simulink model "preliminar"  (R2013b) (continued)
== system preliminar == (continued)
      mask init:
      | pipeline_init(gcb,'latency',latency);
      settings: AttributesFormatString=Latency = %<latency>
      mask Display:
      | port_label('input', 1, 'd');
      | port_label('output', 1, 'q');
      | disp(['Z^-', num2str(latency)]);
  pipeline35 : Subsystem  AncestorBlock=casper_library_delays/pipeline  mask(pipeline){latency=1}
      mask help:
      | An explicitly laid-out delay line to avoid mapping to SRL16s
      | for use in pipelining to help achieve timing closure.
      mask init:
      | pipeline_init(gcb,'latency',latency);
      settings: AttributesFormatString=Latency = %<latency>
      mask Display:
      | port_label('input', 1, 'd');
      | port_label('output', 1, 'q');
      | disp(['Z^-', num2str(latency)]);
  pipeline36 : Subsystem  AncestorBlock=casper_library_delays/pipeline  mask(pipeline){latency=1}
      mask help:
      | An explicitly laid-out delay line to avoid mapping to SRL16s
      | for use in pipelining to help achieve timing closure.
      mask init:
      | pipeline_init(gcb,'latency',latency);
      settings: AttributesFormatString=Latency = %<latency>
      mask Display:
      | port_label('input', 1, 'd');
      | port_label('output', 1, 'q');
      | disp(['Z^-', num2str(latency)]);
  pipeline37 : Subsystem  AncestorBlock=casper_library_delays/pipeline  mask(pipeline){latency=1}
      mask help:
      | An explicitly laid-out delay line to avoid mapping to SRL16s
      | for use in pipelining to help achieve timing closure.
      mask init:
      | pipeline_init(gcb,'latency',latency);
      settings: AttributesFormatString=Latency = %<latency>
      mask Display:
      | port_label('input', 1, 'd');
      | port_label('output', 1, 'q');
      | disp(['Z^-', num2str(latency)]);
  pipeline38 : Subsystem  AncestorBlock=casper_library_delays/pipeline  mask(pipeline){latency=1}
      mask help:
      | An explicitly laid-out delay line to avoid mapping to SRL16s
      | for use in pipelining to help achieve timing closure.
      mask init:
      | pipeline_init(gcb,'latency',latency);
      settings: AttributesFormatString=Latency = %<latency>
      mask Display:
      | port_label('input', 1, 'd');
      | port_label('output', 1, 'q');
      | disp(['Z^-', num2str(latency)]);
  pipeline39 : Subsystem  AncestorBlock=casper_library_delays/pipeline  mask(pipeline){latency=1}
      mask help:
      | An explicitly laid-out delay line to avoid mapping to SRL16s
      | for use in pipelining to help achieve timing closure.
      mask init:
      | pipeline_init(gcb,'latency',latency);
      settings: AttributesFormatString=Latency = %<latency>
      mask Display:
      | port_label('input', 1, 'd');
      | port_label('output', 1, 'q');
      | disp(['Z^-', num2str(latency)]);
  pipeline40 : Subsystem  AncestorBlock=casper_library_delays/pipeline  mask(pipeline){latency=1}
      mask help:
      | An explicitly laid-out delay line to avoid mapping to SRL16s
      | for use in pipelining to help achieve timing closure.
      mask init:
      | pipeline_init(gcb,'latency',latency);
      settings: AttributesFormatString=Latency = %<latency>
      mask Display:
      | port_label('input', 1, 'd');
      | port_label('output', 1, 'q');
      | disp(['Z^-', num2str(latency)]);
  pipeline41 : Subsystem  AncestorBlock=casper_library_delays/pipeline  mask(pipeline){latency=1}
      mask help:
      | An explicitly laid-out delay line to avoid mapping to SRL16s
      | for use in pipelining to help achieve timing closure.
      mask init:
      | pipeline_init(gcb,'latency',latency);
      settings: AttributesFormatString=Latency = %<latency>
      mask Display:
      | port_label('input', 1, 'd');
      | port_label('output', 1, 'q');
      | disp(['Z^-', num2str(latency)]);
  pipeline42 : Subsystem  AncestorBlock=casper_library_delays/pipeline  mask(pipeline){latency=1}
      mask help:
      | An explicitly laid-out delay line to avoid mapping to SRL16s
      | for use in pipelining to help achieve timing closure.
      mask init:
      | pipeline_init(gcb,'latency',latency);
      settings: AttributesFormatString=Latency = %<latency>
      mask Display:
      | port_label('input', 1, 'd');
      | port_label('output', 1, 'q');
      | disp(['Z^-', num2str(latency)]);
  pipeline43 : Subsystem  AncestorBlock=casper_library_delays/pipeline  mask(pipeline){latency=1}
      mask help:
      | An explicitly laid-out delay line to avoid mapping to SRL16s
      | for use in pipelining to help achieve timing closure.
      mask init:
      | pipeline_init(gcb,'latency',latency);
      settings: AttributesFormatString=Latency = %<latency>
      mask Display:
      | port_label('input', 1, 'd');
      | port_label('output', 1, 'q');
      | disp(['Z^-', num2str(latency)]);
  pipeline44 : Subsystem  AncestorBlock=casper_library_delays/pipeline  mask(pipeline){latency=1}
      mask help:
      | An explicitly laid-out delay line to avoid mapping to SRL16s
      | for use in pipelining to help achieve timing closure.
      mask init:
      | pipeline_init(gcb,'latency',latency);
      settings: AttributesFormatString=Latency = %<latency>
      mask Display:
      | port_label('input', 1, 'd');
      | port_label('output', 1, 'q');
      | disp(['Z^-', num2str(latency)]);
  pipeline56 : Subsystem  AncestorBlock=casper_library_delays/pipeline  mask(pipeline){latency=2}
      mask help:
      | An explicitly laid-out delay line to avoid mapping to SRL16s
      | for use in pipelining to help achieve timing closure.
      mask init:
      | pipeline_init(gcb,'latency',latency);
      settings: AttributesFormatString=Latency = %<latency>
      mask Display:
      | port_label('input', 1, 'd');
      | port_label('output', 1, 'q');
      | disp(['Z^-', num2str(latency)]);
  pipeline57 : Subsystem  AncestorBlock=casper_library_delays/pipeline  mask(pipeline){latency=2}
      mask help:
      | An explicitly laid-out delay line to avoid mapping to SRL16s
      | for use in pipelining to help achieve timing closure.
      mask init:
      | pipeline_init(gcb,'latency',latency);
      settings: AttributesFormatString=Latency = %<latency>
      mask Display:
      | port_label('input', 1, 'd');
      | port_label('output', 1, 'q');
      | disp(['Z^-', num2str(latency)]);
  pipeline58 : Subsystem  AncestorBlock=casper_library_delays/pipeline  mask(pipeline){latency=1}
      mask help:
      | An explicitly laid-out delay line to avoid mapping to SRL16s
      | for use in pipelining to help achieve timing closure.
      mask init:
      | pipeline_init(gcb,'latency',latency);
      settings: AttributesFormatString=Latency = %<latency>
      mask Display:
      | port_label('input', 1, 'd');
      | port_label('output', 1, 'q');
      | disp(['Z^-', num2str(latency)]);
  pipeline59 : Subsystem  AncestorBlock=casper_library_delays/pipeline  mask(pipeline){latency=1}
      mask help:
      | An explicitly laid-out delay line to avoid mapping to SRL16s
      | for use in pipelining to help achieve timing closure.
      mask init:
      | pipeline_init(gcb,'latency',latency);
      settings: AttributesFormatString=Latency = %<latency>
      mask Display:
      | port_label('input', 1, 'd');
      | port_label('output', 1, 'q');
      | disp(['Z^-', num2str(latency)]);
  pipeline60 : Subsystem  AncestorBlock=casper_library_delays/pipeline  mask(pipeline){latency=1}
      mask help:
      | An explicitly laid-out delay line to avoid mapping to SRL16s
      | for use in pipelining to help achieve timing closure.
      mask init:
      | pipeline_init(gcb,'latency',latency);
      settings: AttributesFormatString=Latency = %<latency>
      mask Display:
      | port_label('input', 1, 'd');
      | port_label('output', 1, 'q');
      | disp(['Z^-', num2str(latency)]);
  pipeline61 : Subsystem  AncestorBlock=casper_library_delays/pipeline  mask(pipeline){latency=2}
      mask help:
      | An explicitly laid-out delay line to avoid mapping to SRL16s
      | for use in pipelining to help achieve timing closure.
      mask init:
      | pipeline_init(gcb,'latency',latency);
      settings: AttributesFormatString=Latency = %<latency>
      mask Display:
      | port_label('input', 1, 'd');
      | port_label('output', 1, 'q');
      | disp(['Z^-', num2str(latency)]);
  pipeline62 : Subsystem  AncestorBlock=casper_library_delays/pipeline  mask(pipeline){latency=2}
      mask help:
      | An explicitly laid-out delay line to avoid mapping to SRL16s
      | for use in pipelining to help achieve timing closure.
      mask init:
      | pipeline_init(gcb,'latency',latency);
      settings: AttributesFormatString=Latency = %<latency>
      mask Display:
      | port_label('input', 1, 'd');
      | port_label('output', 1, 'q');
      | disp(['Z^-', num2str(latency)]);
  pipeline63 : Subsystem  AncestorBlock=casper_library_delays/pipeline  mask(pipeline){latency=1}
      mask help:
      | An explicitly laid-out delay line to avoid mapping to SRL16s
      | for use in pipelining to help achieve timing closure.
      mask init:
      | pipeline_init(gcb,'latency',latency);
      settings: AttributesFormatString=Latency = %<latency>
      mask Display:
      | port_label('input', 1, 'd');
      | port_label('output', 1, 'q');
      | disp(['Z^-', num2str(latency)]);
  pipeline64 : Subsystem  AncestorBlock=casper_library_delays/pipeline  mask(pipeline){latency=1}
      mask help:
      | An explicitly laid-out delay line to avoid mapping to SRL16s
      | for use in pipelining to help achieve timing closure.
      mask init:
      | pipeline_init(gcb,'latency',latency);
      settings: AttributesFormatString=Latency = %<latency>
      mask Display:
      | port_label('input', 1, 'd');
      | port_label('output', 1, 'q');
      | disp(['Z^-', num2str(latency)]);
  pipeline65 : Subsystem  AncestorBlock=casper_library_delays/pipeline  mask(pipeline){latency=2}
      mask help:
      | An explicitly laid-out delay line to avoid mapping to SRL16s
      | for use in pipelining to help achieve timing closure.
      mask init:
      | pipeline_init(gcb,'latency',latency);
      settings: AttributesFormatString=Latency = %<latency>
      mask Display:
      | port_label('input', 1, 'd');
      | port_label('output', 1, 'q');
      | disp(['Z^-', num2str(latency)]);
  pipeline66 : Subsystem  AncestorBlock=casper_library_delays/pipeline  mask(pipeline){latency=2}
      mask help:
      | An explicitly laid-out delay line to avoid mapping to SRL16s
      | for use in pipelining to help achieve timing closure.
      mask init:
      | pipeline_init(gcb,'latency',latency);
      settings: AttributesFormatString=Latency = %<latency>
      mask Display:
      | port_label('input', 1, 'd');
      | port_label('output', 1, 'q');
      | disp(['Z^-', num2str(latency)]);
  pipeline67 : Subsystem  AncestorBlock=casper_library_delays/pipeline  mask(pipeline){latency=1}
      mask help:
      | An explicitly laid-out delay line to avoid mapping to SRL16s
      | for use in pipelining to help achieve timing closure.
      mask init:
      | pipeline_init(gcb,'latency',latency);
      settings: AttributesFormatString=Latency = %<latency>
      mask Display:
      | port_label('input', 1, 'd');
      | port_label('output', 1, 'q');
      | disp(['Z^-', num2str(latency)]);
  pipeline68 : Subsystem  AncestorBlock=casper_library_delays/pipeline  mask(pipeline){latency=1}
      mask help:
      | An explicitly laid-out delay line to avoid mapping to SRL16s
      | for use in pipelining to help achieve timing closure.
      mask init:
      | pipeline_init(gcb,'latency',latency);
      settings: AttributesFormatString=Latency = %<latency>
      mask Display:
      | port_label('input', 1, 'd');
      | port_label('output', 1, 'q');
      | disp(['Z^-', num2str(latency)]);
  pipeline69 : Subsystem  AncestorBlock=casper_library_delays/pipeline  mask(pipeline){latency=2}
      mask help:
      | An explicitly laid-out delay line to avoid mapping to SRL16s
      | for use in pipelining to help achieve timing closure.
      mask init:
      | pipeline_init(gcb,'latency',latency);
      settings: AttributesFormatString=Latency = %<latency>
      mask Display:
      | port_label('input', 1, 'd');
      | port_label('output', 1, 'q');
      | disp(['Z^-', num2str(latency)]);
  pipeline70 : Subsystem  AncestorBlock=casper_library_delays/pipeline  mask(pipeline){latency=2}
      mask help:
      | An explicitly laid-out delay line to avoid mapping to SRL16s
      | for use in pipelining to help achieve timing closure.
      mask init:
      | pipeline_init(gcb,'latency',latency);
      settings: AttributesFormatString=Latency = %<latency>
      mask Display:
      | port_label('input', 1, 'd');
      | port_label('output', 1, 'q');
      | disp(['Z^-', num2str(latency)]);
  pipeline71 : Subsystem  AncestorBlock=casper_library_delays/pipeline  mask(pipeline){latency=1}
      mask help:
      | An explicitly laid-out delay line to avoid mapping to SRL16s
      | for use in pipelining to help achieve timing closure.
      mask init:
      | pipeline_init(gcb,'latency',latency);
      settings: AttributesFormatString=Latency = %<latency>
      mask Display:
      | port_label('input', 1, 'd');
      | port_label('output', 1, 'q');
      | disp(['Z^-', num2str(latency)]);
  pipeline72 : Subsystem  AncestorBlock=casper_library_delays/pipeline  mask(pipeline){latency=5}
      mask help:
      | An explicitly laid-out delay line to avoid mapping to SRL16s
      | for use in pipelining to help achieve timing closure.
      mask init:
      | pipeline_init(gcb,'latency',latency);
      settings: AttributesFormatString=Latency = %<latency>
      mask Display:
      | port_label('input', 1, 'd');
      | port_label('output', 1, 'q');
      | disp(['Z^-', num2str(latency)]);
  pipeline73 : Subsystem  AncestorBlock=casper_library_delays/pipeline  mask(pipeline){latency=1}
      mask help:
      | An explicitly laid-out delay line to avoid mapping to SRL16s
      | for use in pipelining to help achieve timing closure.
      mask init:
      | pipeline_init(gcb,'latency',latency);
      settings: AttributesFormatString=Latency = %<latency>
      mask Display:
      | port_label('input', 1, 'd');
      | port_label('output', 1, 'q');
      | disp(['Z^-', num2str(latency)]);
  pipeline74 : Subsystem  AncestorBlock=casper_library_delays/pipeline  mask(pipeline){latency=2}
      mask help:
      | An explicitly laid-out delay line to avoid mapping to SRL16s
      | for use in pipelining to help achieve timing closure.
      mask init:
      | pipeline_init(gcb,'latency',latency);
      settings: AttributesFormatString=Latency = %<latency>
      mask Display:
      | port_label('input', 1, 'd');
      | port_label('output', 1, 'q');
      | disp(['Z^-', num2str(latency)]);
  pipeline75 : Subsystem  AncestorBlock=casper_library_delays/pipeline  mask(pipeline){latency=2}
      mask help:
      | An explicitly laid-out delay line to avoid mapping to SRL16s
      | for use in pipelining to help achieve timing closure.
      mask init:
      | pipeline_init(gcb,'latency',latency);
      settings: AttributesFormatString=Latency = %<latency>
      mask Display:
      | port_label('input', 1, 'd');
      | port_label('output', 1, 'q');
      | disp(['Z^-', num2str(latency)]);
  pipeline76 : Subsystem  AncestorBlock=casper_library_delays/pipeline  mask(pipeline){latency=1}
      mask help:
      | An explicitly laid-out delay line to avoid mapping to SRL16s
      | for use in pipelining to help achieve timing closure.
      mask init:
      | pipeline_init(gcb,'latency',latency);
      settings: AttributesFormatString=Latency = %<latency>
      mask Display:
      | port_label('input', 1, 'd');
      | port_label('output', 1, 'q');
      | disp(['Z^-', num2str(latency)]);
  pipeline77 : Subsystem  AncestorBlock=casper_library_delays/pipeline  mask(pipeline){latency=1}
      mask help:
      | An explicitly laid-out delay line to avoid mapping to SRL16s
      | for use in pipelining to help achieve timing closure.
      mask init:
      | pipeline_init(gcb,'latency',latency);
      settings: AttributesFormatString=Latency = %<latency>
      mask Display:
      | port_label('input', 1, 'd');
      | port_label('output', 1, 'q');
      | disp(['Z^-', num2str(latency)]);
  pipeline78 : Subsystem  AncestorBlock=casper_library_delays/pipeline  mask(pipeline){latency=1}
      mask help:
      | An explicitly laid-out delay line to avoid mapping to SRL16s
      | for use in pipelining to help achieve timing closure.
      mask init:
      | pipeline_init(gcb,'latency',latency);
      settings: AttributesFormatString=Latency = %<latency>
      mask Display:
      | port_label('input', 1, 'd');
      | port_label('output', 1, 'q');
      | disp(['Z^-', num2str(latency)]);
  pipeline79 : Subsystem  AncestorBlock=casper_library_delays/pipeline  mask(pipeline){latency=2}
      mask help:
      | An explicitly laid-out delay line to avoid mapping to SRL16s
      | for use in pipelining to help achieve timing closure.
      mask init:
      | pipeline_init(gcb,'latency',latency);
      settings: AttributesFormatString=Latency = %<latency>
      mask Display:
      | port_label('input', 1, 'd');
      | port_label('output', 1, 'q');
      | disp(['Z^-', num2str(latency)]);
  pipeline8 : Subsystem  AncestorBlock=casper_library_delays/pipeline  mask(pipeline){latency=1}
      mask help:
      | An explicitly laid-out delay line to avoid mapping to SRL16s
      | for use in pipelining to help achieve timing closure.
      mask init:
      | pipeline_init(gcb,'latency',latency);
      settings: AttributesFormatString=Latency = %<latency>
      mask Display:
      | port_label('input', 1, 'd');
      | port_label('output', 1, 'q');
      | disp(['Z^-', num2str(latency)]);
  pipeline80 : Subsystem  AncestorBlock=casper_library_delays/pipeline  mask(pipeline){latency=2}
      mask help:
      | An explicitly laid-out delay line to avoid mapping to SRL16s
      | for use in pipelining to help achieve timing closure.
      mask init:
      | pipeline_init(gcb,'latency',latency);
      settings: AttributesFormatString=Latency = %<latency>
      mask Display:
      | port_label('input', 1, 'd');
      | port_label('output', 1, 'q');
      | disp(['Z^-', num2str(latency)]);
  pipeline81 : Subsystem  AncestorBlock=casper_library_delays/pipeline  mask(pipeline){latency=1}
      mask help:
      | An explicitly laid-out delay line to avoid mapping to SRL16s
      | for use in pipelining to help achieve timing closure.
      mask init:
      | pipeline_init(gcb,'latency',latency);
      settings: AttributesFormatString=Latency = %<latency>
      mask Display:
      | port_label('input', 1, 'd');
      | port_label('output', 1, 'q');
      | disp(['Z^-', num2str(latency)]);
  pipeline82 : Subsystem  AncestorBlock=casper_library_delays/pipeline  mask(pipeline){latency=1}
      mask help:
      | An explicitly laid-out delay line to avoid mapping to SRL16s
      | for use in pipelining to help achieve timing closure.
      mask init:
      | pipeline_init(gcb,'latency',latency);
      settings: AttributesFormatString=Latency = %<latency>
      mask Display:
      | port_label('input', 1, 'd');
      | port_label('output', 1, 'q');
      | disp(['Z^-', num2str(latency)]);
  pipeline83 : Subsystem  AncestorBlock=casper_library_delays/pipeline  mask(pipeline){latency=2}
      mask help:
      | An explicitly laid-out delay line to avoid mapping to SRL16s
      | for use in pipelining to help achieve timing closure.
      mask init:
      | pipeline_init(gcb,'latency',latency);
      settings: AttributesFormatString=Latency = %<latency>
      mask Display:
      | port_label('input', 1, 'd');
      | port_label('output', 1, 'q');
      | disp(['Z^-', num2str(latency)]);
  pipeline84 : Subsystem  AncestorBlock=casper_library_delays/pipeline  mask(pipeline){latency=2}
      mask help:
      | An explicitly laid-out delay line to avoid mapping to SRL16s
      | for use in pipelining to help achieve timing closure.
      mask init:
      | pipeline_init(gcb,'latency',latency);
      settings: AttributesFormatString=Latency = %<latency>
      mask Display:
      | port_label('input', 1, 'd');
      | port_label('output', 1, 'q');
      | disp(['Z^-', num2str(latency)]);
  pipeline85 : Subsystem  AncestorBlock=casper_library_delays/pipeline  mask(pipeline){latency=1}
      mask help:
      | An explicitly laid-out delay line to avoid mapping to SRL16s
      | for use in pipelining to help achieve timing closure.
      mask init:
      | pipeline_init(gcb,'latency',latency);
      settings: AttributesFormatString=Latency = %<latency>
      mask Display:
      | port_label('input', 1, 'd');
      | port_label('output', 1, 'q');
      | disp(['Z^-', num2str(latency)]);
  pipeline86 : Subsystem  AncestorBlock=casper_library_delays/pipeline  mask(pipeline){latency=1}
      mask help:
      | An explicitly laid-out delay line to avoid mapping to SRL16s
      | for use in pipelining to help achieve timing closure.
      mask init:
      | pipeline_init(gcb,'latency',latency);
      settings: AttributesFormatString=Latency = %<latency>
      mask Display:
      | port_label('input', 1, 'd');
      | port_label('output', 1, 'q');
      | disp(['Z^-', num2str(latency)]);
  pipeline87 : Subsystem  AncestorBlock=casper_library_delays/pipeline  mask(pipeline){latency=2}
      mask help:
      | An explicitly laid-out delay line to avoid mapping to SRL16s
      | for use in pipelining to help achieve timing closure.
      mask init:
      | pipeline_init(gcb,'latency',latency);
      settings: AttributesFormatString=Latency = %<latency>
      mask Display:
      | port_label('input', 1, 'd');
      | port_label('output', 1, 'q');
      | disp(['Z^-', num2str(latency)]);
  pipeline88 : Subsystem  AncestorBlock=casper_library_delays/pipeline  mask(pipeline){latency=2}
      mask help:
      | An explicitly laid-out delay line to avoid mapping to SRL16s
      | for use in pipelining to help achieve timing closure.
      mask init:
      | pipeline_init(gcb,'latency',latency);
      settings: AttributesFormatString=Latency = %<latency>
      mask Display:
      | port_label('input', 1, 'd');
      | port_label('output', 1, 'q');
      | disp(['Z^-', num2str(latency)]);
  pipeline89 : Subsystem  AncestorBlock=casper_library_delays/pipeline  mask(pipeline){latency=1}
      mask help:
      | An explicitly laid-out delay line to avoid mapping to SRL16s
      | for use in pipelining to help achieve timing closure.
      mask init:
      | pipeline_init(gcb,'latency',latency);
      settings: AttributesFormatString=Latency = %<latency>
      mask Display:
      | port_label('input', 1, 'd');
      | port_label('output', 1, 'q');
      | disp(['Z^-', num2str(latency)]);
  pipeline90 : Subsystem  AncestorBlock=casper_library_delays/pipeline  mask(pipeline){latency=5}
      mask help:
      | An explicitly laid-out delay line to avoid mapping to SRL16s
      | for use in pipelining to help achieve timing closure.
      mask init:
      | pipeline_init(gcb,'latency',latency);
      settings: AttributesFormatString=Latency = %<latency>
      mask Display:
      | port_label('input', 1, 'd');
      | port_label('output', 1, 'q');
      | disp(['Z^-', num2str(latency)]);
  pipeline91 : Subsystem  AncestorBlock=casper_library_delays/pipeline  mask(pipeline){latency=1}
      mask help:
      | An explicitly laid-out delay line to avoid mapping to SRL16s
      | for use in pipelining to help achieve timing closure.
      mask init:
      | pipeline_init(gcb,'latency',latency);
      settings: AttributesFormatString=Latency = %<latency>
      mask Display:
      | port_label('input', 1, 'd');
      | port_label('output', 1, 'q');
      | disp(['Z^-', num2str(latency)]);
  pipeline92 : Subsystem  AncestorBlock=casper_library_delays/pipeline  mask(pipeline){latency=2}
      mask help:
      | An explicitly laid-out delay line to avoid mapping to SRL16s
      | for use in pipelining to help achieve timing closure.
      mask init:
      | pipeline_init(gcb,'latency',latency);
      settings: AttributesFormatString=Latency = %<latency>
      mask Display:
      | port_label('input', 1, 'd');
      | port_label('output', 1, 'q');
      | disp(['Z^-', num2str(latency)]);
  power{1..7,16} (8 alike) : casper_library_misc/power  BitWidth=18  add_latency=2  mult_latency=3
  power_convert0 : Subsystem  mask{shift_val=13, conv_width=20, conv_point=19}
      mask prompts: shift_val "shift"; conv_width "convert width"; conv_point "convert point"
  power_convert1 : Subsystem  mask{shift_val=13, conv_width=20, conv_point=19}
      mask prompts: shift_val "shift"; conv_width "convert width"; conv_point "convert point"
  r11 : Subsystem  Tag=xps:bram  AncestorBlock=xps_library/Shared_BRAM  mask{arith_type=Unsigned, addr_width=9, data_width=128, reg_prim_output=off, reg_core_output=off, optimization=Minimum_Area, data_bin_pt=0, init_vals=[0:2^10-1], sample_rate=1}
      mask prompts: arith_type "Output Data Type"; addr_width "Address width"; data_width "Data Width"; reg_prim_output "Register Primitive Output"; reg_core_output "Register Core Output"; data_bin_pt "Data Binary Point"; init_vals "Initial values (simulation only)"; sample_rate "Sample rate"
      mask init:
      | shared_bram_mask;
      mask TypeOptions arith_type: Unsigned | Signed  (2's comp)
      mask TypeOptions data_width: 8 | 16 | 32 | 64 | 128
      mask TypeOptions optimization: Minimum_Area | Low_Power
  r12 : Subsystem  Tag=xps:bram  AncestorBlock=xps_library/Shared_BRAM  mask{arith_type=Unsigned, addr_width=9, data_width=128, reg_prim_output=off, reg_core_output=off, optimization=Minimum_Area, data_bin_pt=0, init_vals=[0:2^10-1], sample_rate=1}
      mask prompts: arith_type "Output Data Type"; addr_width "Address width"; data_width "Data Width"; reg_prim_output "Register Primitive Output"; reg_core_output "Register Core Output"; data_bin_pt "Data Binary Point"; init_vals "Initial values (simulation only)"; sample_rate "Sample rate"
      mask init:
      | shared_bram_mask;
      mask TypeOptions arith_type: Unsigned | Signed  (2's comp)
      mask TypeOptions data_width: 8 | 16 | 32 | 64 | 128
      mask TypeOptions optimization: Minimum_Area | Low_Power
  r22 : xps_library/Shared_BRAM  Tag=xps:bram  arith_type=Unsigned  addr_width=10  data_width=32  reg_prim_output=off  reg_core_output=off  optimization=Minimum_Area  data_bin_pt=0  init_vals=[0:2^10-1]  sample_rate=1
  simple_bram_vacc_0_0 : Subsystem  AncestorBlock=casper_library_accumulators/simple_bram_vacc  mask(simple_bram_vacc){vec_len=512, arith_type=Unsigned, n_bits=32, bin_pt=19}
      mask prompts: vec_len "Vector length:"; arith_type "Output type:"; n_bits "Bit width (output):"; bin_pt "Binary point (output):"
      mask help: This simple vector accumulator is based on a shift register. It outputs its previous accumulated vector after a new_acc pulse is received.
      mask init:
      | simple_bram_vacc_init(gcb, ...
      |     'vec_len', vec_len, ...
      |     'arith_type', arith_type, ...
      |     'n_bits', n_bits, ...
      |     'bin_pt', bin_pt);
      mask TypeOptions arith_type: Signed | Unsigned
  simple_bram_vacc_0_1 : Subsystem  AncestorBlock=casper_library_accumulators/simple_bram_vacc  mask(simple_bram_vacc){vec_len=512, arith_type=Unsigned, n_bits=32, bin_pt=19}
      mask prompts: vec_len "Vector length:"; arith_type "Output type:"; n_bits "Bit width (output):"; bin_pt "Binary point (output):"
      mask help: This simple vector accumulator is based on a shift register. It outputs its previous accumulated vector after a new_acc pulse is received.
      mask init:
      | simple_bram_vacc_init(gcb, ...
      |     'vec_len', vec_len, ...
      |     'arith_type', arith_type, ...
      |     'n_bits', n_bits, ...
      |     'bin_pt', bin_pt);
      mask TypeOptions arith_type: Signed | Unsigned
  simple_bram_vacc_0_2 : Subsystem  AncestorBlock=casper_library_accumulators/simple_bram_vacc  mask(simple_bram_vacc){vec_len=512, arith_type=Unsigned, n_bits=32, bin_pt=19}
      mask prompts: vec_len "Vector length:"; arith_type "Output type:"; n_bits "Bit width (output):"; bin_pt "Binary point (output):"
      mask help: This simple vector accumulator is based on a shift register. It outputs its previous accumulated vector after a new_acc pulse is received.
      mask init:
      | simple_bram_vacc_init(gcb, ...
      |     'vec_len', vec_len, ...
      |     'arith_type', arith_type, ...
      |     'n_bits', n_bits, ...
      |     'bin_pt', bin_pt);
      mask TypeOptions arith_type: Signed | Unsigned
  simple_bram_vacc_0_3 : Subsystem  AncestorBlock=casper_library_accumulators/simple_bram_vacc  mask(simple_bram_vacc){vec_len=512, arith_type=Unsigned, n_bits=32, bin_pt=19}
      mask prompts: vec_len "Vector length:"; arith_type "Output type:"; n_bits "Bit width (output):"; bin_pt "Binary point (output):"
      mask help: This simple vector accumulator is based on a shift register. It outputs its previous accumulated vector after a new_acc pulse is received.
      mask init:
      | simple_bram_vacc_init(gcb, ...
      |     'vec_len', vec_len, ...
      |     'arith_type', arith_type, ...
      |     'n_bits', n_bits, ...
      |     'bin_pt', bin_pt);
      mask TypeOptions arith_type: Signed | Unsigned
  simple_bram_vacc_0_4 : Subsystem  AncestorBlock=casper_library_accumulators/simple_bram_vacc  mask(simple_bram_vacc){vec_len=512, arith_type=Unsigned, n_bits=32, bin_pt=19}
      mask prompts: vec_len "Vector length:"; arith_type "Output type:"; n_bits "Bit width (output):"; bin_pt "Binary point (output):"
      mask help: This simple vector accumulator is based on a shift register. It outputs its previous accumulated vector after a new_acc pulse is received.
      mask init:
      | simple_bram_vacc_init(gcb, ...
      |     'vec_len', vec_len, ...
      |     'arith_type', arith_type, ...
      |     'n_bits', n_bits, ...
      |     'bin_pt', bin_pt);
      mask TypeOptions arith_type: Signed | Unsigned
  simple_bram_vacc_0_5 : Subsystem  AncestorBlock=casper_library_accumulators/simple_bram_vacc  mask(simple_bram_vacc){vec_len=512, arith_type=Unsigned, n_bits=32, bin_pt=19}
      mask prompts: vec_len "Vector length:"; arith_type "Output type:"; n_bits "Bit width (output):"; bin_pt "Binary point (output):"
      mask help: This simple vector accumulator is based on a shift register. It outputs its previous accumulated vector after a new_acc pulse is received.
      mask init:
      | simple_bram_vacc_init(gcb, ...
      |     'vec_len', vec_len, ...
      |     'arith_type', arith_type, ...
      |     'n_bits', n_bits, ...
      |     'bin_pt', bin_pt);
      mask TypeOptions arith_type: Signed | Unsigned
  simple_bram_vacc_0_6 : Subsystem  AncestorBlock=casper_library_accumulators/simple_bram_vacc  mask(simple_bram_vacc){vec_len=512, arith_type=Unsigned, n_bits=32, bin_pt=19}
      mask prompts: vec_len "Vector length:"; arith_type "Output type:"; n_bits "Bit width (output):"; bin_pt "Binary point (output):"
      mask help: This simple vector accumulator is based on a shift register. It outputs its previous accumulated vector after a new_acc pulse is received.
      mask init:
      | simple_bram_vacc_init(gcb, ...
      |     'vec_len', vec_len, ...
      |     'arith_type', arith_type, ...
      |     'n_bits', n_bits, ...
      |     'bin_pt', bin_pt);
      mask TypeOptions arith_type: Signed | Unsigned
  simple_bram_vacc_0_7 : Subsystem  AncestorBlock=casper_library_accumulators/simple_bram_vacc  mask(simple_bram_vacc){vec_len=512, arith_type=Unsigned, n_bits=32, bin_pt=19}
      mask prompts: vec_len "Vector length:"; arith_type "Output type:"; n_bits "Bit width (output):"; bin_pt "Binary point (output):"
      mask help: This simple vector accumulator is based on a shift register. It outputs its previous accumulated vector after a new_acc pulse is received.
      mask init:
      | simple_bram_vacc_init(gcb, ...
      |     'vec_len', vec_len, ...
      |     'arith_type', arith_type, ...
      |     'n_bits', n_bits, ...
      |     'bin_pt', bin_pt);
      mask TypeOptions arith_type: Signed | Unsigned
  snap_trig : Subsystem  Tag=xps:sw_reg  AncestorBlock=xps_library/software_register  mask(swreg){io_dir=From Processor, io_delay=0, sample_period=1, names=reg, bitwidths=32, bin_pts=0, arith_types=2, sim_port=off, show_format=off}
      mask prompts: io_dir "I/O direction"; io_delay "I/O delay"; sample_period "Sample period"; names "Bitfield names [msb...lsb]"; bitwidths "Bitfield widths"; bin_pts "Bitfield binary pts"; arith_types "Bitfield types, ufix=0, fix=1, bool=2"; sim_port "Provide sim input/output?"; show_format "Print format string?"
      mask help: A 32-bit software-accessible register. Can be divided into bitfields of varying widths and types using the fields in the block mask.
      mask init:
      | try
      |     swreg_init(gcb)
      | catch ex
      |     disp(sprintf('%s: %s', gcb, ex.message))
      |     errordlg(ex.message)
      | end
      settings: AttributesFormatString=1input
      mask TypeOptions io_dir: To Processor | From Processor
      mask Callback io_dir:
      | mask_enables = get_param(gcb, 'MaskEnables');
      | mask_names = get_param(gcb, 'MaskNames');
      | if strcmp(get_param(gcb, 'io_dir'), 'To Processor'),
      |     mask_enables{ismember(mask_names, 'sample_period')} = 'off';
      | else
      |     mask_enables{ismember(mask_names, 'sample_period')} = 'on';
      | end
      | set_param(gcb, 'MaskEnables', mask_enables);
  sync_gen : Subsystem  AncestorBlock=casper_library_misc/sync_gen  mask(sync_gen){sim_acc_len=1, fft_size=8192, fft_simult_inputs=8, pfb_fir_taps=3, reorder_vec=[2,2,5], scale=1}
      mask prompts: sim_acc_len "Simulation Accumulation Length"; fft_size "FFT Size"; fft_simult_inputs "Simultaneous Inputs (FFT)"; pfb_fir_taps "Taps in pfb_fir (use 1 if no pfb_fir)"; reorder_vec "Reorder Orders (e.g. [2 2 2])"
      mask help:
      | This block computes the sync period based on system parameters as
      | described in Casper memo.  Detailed descritions of the block's
      | variables can be found in the 'Block Documentation' section of
      | the CASPER wiki.
      mask init:
      | sync_gen_init(gcb, ...
      |     'sim_acc_len', sim_acc_len, ...
      |     'fft_size', fft_size, ...
      |     'fft_simult_inputs', fft_simult_inputs,...
      |     'pfb_fir_taps', pfb_fir_taps, ...
      |     'reorder_vec', reorder_vec, ...
      |     'scale', scale);
      settings: AttributesFormatString=sim_sync_period=30720
      mask Help:
      | SyncPeriod=LCM(reorder_vec)*(acc_num)*(pfb_fir_len)*(fft_size/fft_simult_inputs)
      | 
      | See CASPER sync pulse memo for more details.
  wires:
  fft_wideband_real0/6(of) -> Terminator1/1
  bus_create/1(bus_out) -> pipeline276/1
  acc_len/1(in_reg) -> pipeline250/1(d)
  cnt_rst/1(in_reg) -> pipeline251/1(d)
  pipeline250/1(q) -> [acc_len]
  pipeline251/1(q) -> Slice1/1
  Band-Limited White Noise/1 -> asiaa_adc5g0/1(sim_a)
  Constant13/1 -> asiaa_adc5g0/3(sim_sync)
  sync_gen/1(sync_out) -> [sync_out]
  pipeline262/1 -> pfb_fir_real0/8(pol1_in7)
  pipeline257/1 -> pfb_fir_real0/3(pol1_in2)
  pipeline261/1 -> pfb_fir_real0/7(pol1_in6)
  pipeline260/1 -> pfb_fir_real0/6(pol1_in5)
  pipeline259/1 -> pfb_fir_real0/5(pol1_in4)
  pipeline258/1 -> pfb_fir_real0/4(pol1_in3)
  pipeline263/1 -> pfb_fir_real0/9(pol1_in8)
  pipeline256/1 -> pfb_fir_real0/2(pol1_in1)
  pipeline16/1 -> pfb_fir_real0/1(sync)
  pfb_fir_real0/1(sync_out) -> Delay15/1
  Delay15/1 -> fft_wideband_real0/1(sync)
  fft_wideband_real0/1(sync_out) -> pipeline275/1
  fft_wideband_real0/2(out00) -> pipeline1/1
  fft_wideband_real0/3(out01) -> pipeline2/1
  fft_wideband_real0/4(out02) -> pipeline3/1
  fft_wideband_real0/5(out03) -> pipeline9/1
  pipeline276/1 -> adcsnap0/1(din)
  Slice1/1 -> [cnt_rst]
  Band-Limited White Noise2/1 -> asiaa_adc5g0/2(sim_c)
  asiaa_adc5g0/17(sync_out) -> Terminator/1
  asiaa_adc5g0/1(a0) -> conv_bus0/1(i0)
  asiaa_adc5g0/2(a1) -> conv_bus0/2(i1)
  asiaa_adc5g0/3(a2) -> conv_bus0/3(i2)
  asiaa_adc5g0/4(a3) -> conv_bus0/4(i3)
  asiaa_adc5g0/5(a4) -> conv_bus0/5(i4)
  asiaa_adc5g0/6(a5) -> conv_bus0/6(i5)
  asiaa_adc5g0/7(a6) -> conv_bus0/7(i6)
  asiaa_adc5g0/8(a7) -> conv_bus0/8(i7)
  delay_wideband_prog/8(data_out6) -> bus_create/7(in7), pipeline262/1
  delay_wideband_prog/6(data_out4) -> bus_create/5(in5), pipeline260/1
  delay_wideband_prog/5(data_out3) -> bus_create/4(in4), pipeline259/1
  delay_wideband_prog/4(data_out2) -> bus_create/3(in3), pipeline258/1
  delay_wideband_prog/3(data_out1) -> bus_create/2(in2), pipeline257/1
  delay_wideband_prog/2(data_out0) -> bus_create/1(in1), pipeline256/1
  bus_create1/1(bus_out) -> pipeline4/1
  pipeline4/1 -> adcsnap1/1(din)
  delay_wideband_prog/9(data_out7) -> bus_create/8(in8), pipeline263/1
  [cnt_rst] -> acc_cntrl0/3(rst)
  [acc_len] -> acc_cntrl0/1(acc_len)
  dout_0a_0/1(data_out) -> Terminator9/1
  Reinterpret31/1 -> dout_0a_0/2(data_in)
  simple_bram_vacc_0_0/1(dout) -> pipeline74/1(d)
  simple_bram_vacc_0_0/2(valid) -> pipeline56/1(d)
  pipeline73/1(q) -> simple_bram_vacc_0_0/2(din)
  pipeline59/1(q) -> pipeline57/1(d)
  pipeline74/1(q) -> Reinterpret31/1
  pipeline56/1(q) -> Counter1/2, dout_0a_0/3(we)
  pipeline57/1(q) -> Counter1/1
  pipeline58/1(q) -> simple_bram_vacc_0_0/1(new_acc)
  dout_0a_1/1(data_out) -> Terminator6/1
  Reinterpret24/1 -> dout_0a_1/2(data_in)
  simple_bram_vacc_0_1/1(dout) -> pipeline61/1(d)
  simple_bram_vacc_0_1/2(valid) -> pipeline62/1(d)
  pipeline60/1(q) -> simple_bram_vacc_0_1/2(din)
  pipeline61/1(q) -> Reinterpret24/1
  pipeline62/1(q) -> dout_0a_1/3(we)
  pipeline63/1(q) -> simple_bram_vacc_0_1/1(new_acc)
  dout_0a_2/1(data_out) -> Terminator7/1
  Reinterpret25/1 -> dout_0a_2/2(data_in)
  simple_bram_vacc_0_2/1(dout) -> pipeline65/1(d)
  simple_bram_vacc_0_2/2(valid) -> pipeline66/1(d)
  pipeline64/1(q) -> simple_bram_vacc_0_2/2(din)
  pipeline65/1(q) -> Reinterpret25/1
  pipeline66/1(q) -> dout_0a_2/3(we)
  pipeline67/1(q) -> simple_bram_vacc_0_2/1(new_acc)
  dout_0a_3/1(data_out) -> Terminator8/1
  Reinterpret26/1 -> dout_0a_3/2(data_in)
  simple_bram_vacc_0_3/1(dout) -> pipeline69/1(d)
  simple_bram_vacc_0_3/2(valid) -> pipeline70/1(d)
  pipeline68/1(q) -> simple_bram_vacc_0_3/2(din)
  pipeline69/1(q) -> Reinterpret26/1
  pipeline70/1(q) -> dout_0a_3/3(we)
  pipeline71/1(q) -> simple_bram_vacc_0_3/1(new_acc)
  Delay32/1 -> pipeline73/1(d)
  Delay34/1 -> pipeline60/1(d)
  Delay35/1 -> pipeline64/1(d)
  Delay36/1 -> pipeline68/1(d)
  Counter1/1 -> dout_0a_1/1(addr), dout_0a_2/1(addr), dout_0a_3/1(addr), dout_0a_0/1(addr)
  acc_cntrl0/1(new_acc) -> pipeline63/1(d), pipeline71/1(d), pipeline67/1(d), pipeline58/1(d), pipeline59/1(d)
  pipeline275/1 -> fft0_delay/1(sync_in), pipeline72/1(d)
  pipeline1/1 -> power16/1, fft0_delay/2(In1)
  pipeline2/1 -> fft0_delay/3(In2), power1/1
  pipeline3/1 -> fft0_delay/4(In3), power2/1
  pipeline9/1 -> fft0_delay/5(In4), power3/1
  Constant10/1 -> fft_wideband_real1/2(shift)
  fft_wideband_real1/6(of) -> Terminator5/1
  pipeline18/1 -> pfb_fir_real1/8(pol1_in7)
  pipeline11/1 -> pfb_fir_real1/3(pol1_in2)
  pipeline17/1 -> pfb_fir_real1/7(pol1_in6)
  pipeline15/1 -> pfb_fir_real1/6(pol1_in5)
  pipeline14/1 -> pfb_fir_real1/5(pol1_in4)
  pipeline12/1 -> pfb_fir_real1/4(pol1_in3)
  pipeline19/1 -> pfb_fir_real1/9(pol1_in8)
  pipeline10/1 -> pfb_fir_real1/2(pol1_in1)
  pipeline6/1 -> pfb_fir_real1/1(sync)
  pfb_fir_real1/1(sync_out) -> Delay3/1
  Delay3/1 -> fft_wideband_real1/1(sync)
  fft_wideband_real1/1(sync_out) -> pipeline20/1
  fft_wideband_real1/2(out00) -> pipeline5/1
  fft_wideband_real1/3(out01) -> pipeline7/1
  fft_wideband_real1/4(out02) -> pipeline21/1
  fft_wideband_real1/5(out03) -> pipeline93/1
  delay_wideband_prog1/8(data_out6) -> bus_create1/7(in7), pipeline18/1
  delay_wideband_prog1/7(data_out5) -> bus_create1/6(in6), pipeline17/1
  delay_wideband_prog1/6(data_out4) -> bus_create1/5(in5), pipeline15/1
  delay_wideband_prog1/5(data_out3) -> bus_create1/4(in4), pipeline14/1
  delay_wideband_prog1/4(data_out2) -> bus_create1/3(in3), pipeline12/1
  delay_wideband_prog1/3(data_out1) -> bus_create1/2(in2), pipeline11/1
  delay_wideband_prog1/2(data_out0) -> bus_create1/1(in1), pipeline10/1
  delay_wideband_prog1/9(data_out7) -> bus_create1/8(in8), pipeline19/1
  [cnt_rst] -> acc_cntrl1/3(rst)
  [acc_len] -> acc_cntrl1/1(acc_len)
  dout_0c_0/1(data_out) -> Terminator13/1
  Reinterpret4/1 -> dout_0c_0/2(data_in)
  simple_bram_vacc_0_4/1(dout) -> pipeline92/1(d)
  simple_bram_vacc_0_4/2(valid) -> pipeline22/1(d)
  pipeline91/1(q) -> simple_bram_vacc_0_4/2(din)
  pipeline77/1(q) -> pipeline75/1(d)
  pipeline92/1(q) -> Reinterpret4/1
  pipeline22/1(q) -> dout_0c_0/3(we), Counter2/2
  pipeline75/1(q) -> Counter2/1
  pipeline76/1(q) -> simple_bram_vacc_0_4/1(new_acc)
  dout_0c_1/1(data_out) -> Terminator10/1
  Reinterpret1/1 -> dout_0c_1/2(data_in)
  simple_bram_vacc_0_5/1(dout) -> pipeline79/1(d)
  simple_bram_vacc_0_5/2(valid) -> pipeline80/1(d)
  pipeline78/1(q) -> simple_bram_vacc_0_5/2(din)
  pipeline79/1(q) -> Reinterpret1/1
  pipeline80/1(q) -> dout_0c_1/3(we)
  pipeline81/1(q) -> simple_bram_vacc_0_5/1(new_acc)
  dout_0c_2/1(data_out) -> Terminator11/1
  Reinterpret2/1 -> dout_0c_2/2(data_in)
  simple_bram_vacc_0_6/1(dout) -> pipeline83/1(d)
  simple_bram_vacc_0_6/2(valid) -> pipeline84/1(d)
  pipeline82/1(q) -> simple_bram_vacc_0_6/2(din)
  pipeline83/1(q) -> Reinterpret2/1
  pipeline84/1(q) -> dout_0c_2/3(we)
  pipeline85/1(q) -> simple_bram_vacc_0_6/1(new_acc)
  dout_0c_3/1(data_out) -> Terminator12/1
  Reinterpret3/1 -> dout_0c_3/2(data_in)
  simple_bram_vacc_0_7/1(dout) -> pipeline87/1(d)
  simple_bram_vacc_0_7/2(valid) -> pipeline88/1(d)
  pipeline86/1(q) -> simple_bram_vacc_0_7/2(din)
  pipeline87/1(q) -> Reinterpret3/1
  pipeline88/1(q) -> dout_0c_3/3(we)
  pipeline89/1(q) -> simple_bram_vacc_0_7/1(new_acc)
  Delay7/1 -> pipeline91/1(d)
  Delay8/1 -> pipeline78/1(d)
  Delay9/1 -> pipeline82/1(d)
  Delay10/1 -> pipeline86/1(d)
  Counter2/1 -> dout_0c_0/1(addr), dout_0c_1/1(addr), dout_0c_2/1(addr), dout_0c_3/1(addr)
  acc_cntrl1/1(new_acc) -> pipeline77/1(d), pipeline76/1(d), pipeline85/1(d), pipeline89/1(d), pipeline81/1(d)
  pipeline20/1 -> pipeline90/1(d), fft1_delay/1(sync_in)
  pipeline5/1 -> power4/1, fft1_delay/2(In1)
  pipeline7/1 -> fft1_delay/3(In2), power5/1
  pipeline21/1 -> power6/1, fft1_delay/4(In3)
  pipeline93/1 -> fft1_delay/5(In4), power7/1
  delay_wideband_prog/7(data_out5) -> bus_create/6(in6), pipeline261/1
  pfb_fir_real0/9(pol1_out8) -> Delay24/1
  pfb_fir_real0/3(pol1_out2) -> Delay5/1
  pfb_fir_real0/4(pol1_out3) -> Delay16/1
  pfb_fir_real0/5(pol1_out4) -> Delay17/1
  pfb_fir_real0/6(pol1_out5) -> Delay21/1
  pfb_fir_real0/7(pol1_out6) -> Delay22/1
  pfb_fir_real0/8(pol1_out7) -> Delay23/1
  pfb_fir_real0/2(pol1_out1) -> Delay4/1
  pfb_fir_real1/5(pol1_out4) -> Delay28/1
  pfb_fir_real1/6(pol1_out5) -> Delay29/1
  pfb_fir_real1/7(pol1_out6) -> Delay30/1
  pfb_fir_real1/8(pol1_out7) -> Delay31/1
  pfb_fir_real1/9(pol1_out8) -> Delay33/1
  pfb_fir_real1/2(pol1_out1) -> Delay25/1
  pfb_fir_real1/3(pol1_out2) -> Delay26/1
  pfb_fir_real1/4(pol1_out3) -> Delay27/1
  Constant1/1 -> fft_wideband_real0/2(shift)
  Delay4/1 -> fft_wideband_real0/3(in00)
  Delay5/1 -> fft_wideband_real0/4(in01)
  Delay16/1 -> fft_wideband_real0/5(in02)
  Delay17/1 -> fft_wideband_real0/6(in03)
  Delay22/1 -> fft_wideband_real0/8(in05)
  Delay21/1 -> fft_wideband_real0/7(in04)
  Delay24/1 -> fft_wideband_real0/10(in07)
  Delay23/1 -> fft_wideband_real0/9(in06)
  Delay25/1 -> fft_wideband_real1/3(in00)
  Delay26/1 -> fft_wideband_real1/4(in01)
  Delay28/1 -> fft_wideband_real1/6(in03)
  Delay27/1 -> fft_wideband_real1/5(in02)
  Delay29/1 -> fft_wideband_real1/7(in04)
  Delay30/1 -> fft_wideband_real1/8(in05)
  Delay31/1 -> fft_wideband_real1/9(in06)
  Delay33/1 -> fft_wideband_real1/10(in07)
  power16/1 -> power_convert0/1(In1)
  power1/1 -> power_convert0/2(In2)
  power2/1 -> power_convert0/3(In3)
  power3/1 -> power_convert0/4(In4)
  power_convert0/1(Out1) -> Delay32/1
  power_convert0/2(Out2) -> Delay34/1
  power_convert0/3(Out3) -> Delay35/1
  power_convert0/4(Out4) -> Delay36/1
  pipeline72/1(q) -> pipeline127/1(d)
  pipeline127/1(q) -> acc_cntrl0/2(sync)
  power4/1 -> power_convert1/1(In1)
  power5/1 -> power_convert1/2(In2)
  power6/1 -> power_convert1/3(In3)
  power7/1 -> power_convert1/4(In4)
  power_convert1/1(Out1) -> Delay7/1
  power_convert1/2(Out2) -> Delay8/1
  power_convert1/3(Out3) -> Delay9/1
  power_convert1/4(Out4) -> Delay10/1
  pipeline90/1(q) -> pipeline128/1(d)
  pipeline128/1(q) -> acc_cntrl1/2(sync)
  conv_bus0/1(q0) -> delay_wideband_prog/4(data_in0)
  conv_bus0/2(q1) -> delay_wideband_prog/5(data_in1)
  conv_bus0/3(q2) -> delay_wideband_prog/6(data_in2)
  conv_bus0/4(q3) -> delay_wideband_prog/7(data_in3)
  conv_bus0/5(q4) -> delay_wideband_prog/8(data_in4)
  conv_bus0/6(q5) -> delay_wideband_prog/9(data_in5)
  conv_bus0/7(q6) -> delay_wideband_prog/10(data_in6)
  conv_bus0/8(q7) -> delay_wideband_prog/11(data_in7)
  conv_bus1/1(q0) -> delay_wideband_prog1/4(data_in0)
  conv_bus1/2(q1) -> delay_wideband_prog1/5(data_in1)
  conv_bus1/3(q2) -> delay_wideband_prog1/6(data_in2)
  conv_bus1/4(q3) -> delay_wideband_prog1/7(data_in3)
  conv_bus1/5(q4) -> delay_wideband_prog1/8(data_in4)
  conv_bus1/6(q5) -> delay_wideband_prog1/9(data_in5)
  conv_bus1/7(q6) -> delay_wideband_prog1/10(data_in6)
  conv_bus1/8(q7) -> delay_wideband_prog1/11(data_in7)
  asiaa_adc5g0/16(c7) -> conv_bus1/8(i7)
  asiaa_adc5g0/15(c6) -> conv_bus1/7(i6)
  asiaa_adc5g0/14(c5) -> conv_bus1/6(i5)
  asiaa_adc5g0/13(c4) -> conv_bus1/5(i4)
  asiaa_adc5g0/12(c3) -> conv_bus1/4(i3)
  asiaa_adc5g0/11(c2) -> conv_bus1/3(i2)
  asiaa_adc5g0/10(c1) -> conv_bus1/2(i1)
  asiaa_adc5g0/9(c0) -> conv_bus1/1(i0)
  [sync_out] -> delay_wideband_prog1/1(sync)
  delay_wideband_prog1/1(sync_out) -> pipeline6/1
  [sync_out] -> delay_wideband_prog/1(sync)
  delay_wideband_prog/1(sync_out) -> pipeline16/1
  adc0_delay/1(in_reg) -> pipeline8/1(d)
  pipeline8/1(q) -> [adc0_delay]
  adc1_delay/1(in_reg) -> pipeline13/1(d)
  pipeline13/1(q) -> [adc1_delay]
  [adc0_delay] -> delay_wideband_prog/2(delay)
  [adc1_delay] -> delay_wideband_prog1/2(delay)
  Constant/1 -> delay_wideband_prog1/3(ld_delay)
  Constant2/1 -> delay_wideband_prog/3(ld_delay)
  snap_trig/1(in_reg) -> pipeline23/1(d)
  pipeline23/1(q) -> Slice2/1
  Slice2/1 -> [snap_trig]
  [snap_trig] -> adcsnap0/2(we), adcsnap0/3(trig)
  [snap_trig] -> adcsnap1/3(trig), adcsnap1/2(we)
  fft0_delay/2(Out1) -> fft0_conv/2(In1)
  fft0_delay/3(Out2) -> fft0_conv/3(In2)
  fft0_delay/1(sync_out) -> fft0_conv/1(sync_in)
  fft0_delay/4(Out3) -> fft0_conv/4(In3)
  fft0_delay/5(Out4) -> fft0_conv/5(In4)
  fft0_conv/1(sync_out) -> [fft0_sync]
  fft0_conv/2(Out1) -> [fft0_ch0]
  fft0_conv/3(Out2) -> [fft0_ch1]
  fft0_conv/4(Out3) -> [fft0_ch2]
  fft0_conv/5(Out4) -> [fft0_ch3]
  fft1_delay/2(Out1) -> fft1_conv/2(In1)
  fft1_delay/3(Out2) -> fft1_conv/3(In2)
  fft1_delay/1(sync_out) -> fft1_conv/1(sync_in)
  fft1_delay/4(Out3) -> fft1_conv/4(In3)
  fft1_delay/5(Out4) -> fft1_conv/5(In4)
  fft1_conv/5(Out4) -> [fft1_ch3]
  fft1_conv/4(Out3) -> [fft1_ch2]
  fft1_conv/3(Out2) -> [fft1_ch1]
  fft1_conv/2(Out1) -> [fft1_ch0]
  fft1_conv/1(sync_out) -> [fft1_sync]
  [fft0_ch0] -> centrosym0/1(din1)
  [fft1_ch0] -> centrosym0/2(din2)
  [fft0_ch1] -> centrosym1/1(din1)
  [fft1_ch1] -> centrosym1/2(din2)
  [fft0_ch2] -> centrosym2/1(din1)
  [fft1_ch2] -> centrosym2/2(din2)
  [fft0_ch3] -> centrosym3/1(din1)
  [fft1_ch3] -> centrosym3/2(din2)
  Register/1 -> centrosym3/3(din_valid), centrosym2/3(din_valid), centrosym1/3(din_valid), centrosym0/3(din_valid)
  [fft0_sync] -> Register/3, Register/1
  [cnt_rst] -> Register/2
  [fft0_sync] -> pipeline/1(d)
  centrosym0/1(y1_re) -> pipeline24/1(d)
  centrosym0/2(y1_im) -> pipeline25/1(d)
  centrosym0/3(y2_re) -> pipeline26/1(d)
  centrosym0/4(y2_im) -> pipeline27/1(d)
  centrosym0/5(dout_valid) -> pipeline28/1(d)
  pipeline24/1(q) -> correlator0/2(din1_re)
  pipeline25/1(q) -> correlator0/3(din1_im)
  pipeline26/1(q) -> correlator0/4(din2_re)
  pipeline27/1(q) -> correlator0/5(din2_im)
  pipeline28/1(q) -> correlator0/6(din_valid)
  pipeline/1(q) -> acc_cntrl2/2(sync)
  acc_cntrl2/1(new_acc) -> correlator3/1(new_acc), correlator2/1(new_acc), correlator1/1(new_acc), correlator0/1(new_acc)
  pipeline29/1(q) -> correlator1/2(din1_re)
  pipeline32/1(q) -> correlator1/5(din2_im)
  pipeline31/1(q) -> correlator1/4(din2_re)
  pipeline30/1(q) -> correlator1/3(din1_im)
  pipeline33/1(q) -> correlator1/6(din_valid)
  centrosym1/1(y1_re) -> pipeline29/1(d)
  centrosym1/2(y1_im) -> pipeline30/1(d)
  centrosym1/3(y2_re) -> pipeline31/1(d)
  centrosym1/4(y2_im) -> pipeline32/1(d)
  centrosym1/5(dout_valid) -> pipeline33/1(d)
  centrosym2/1(y1_re) -> pipeline34/1(d)
  centrosym2/2(y1_im) -> pipeline35/1(d)
  centrosym2/3(y2_re) -> pipeline36/1(d)
  centrosym2/4(y2_im) -> pipeline37/1(d)
  centrosym2/5(dout_valid) -> pipeline38/1(d)
  pipeline34/1(q) -> correlator2/2(din1_re)
  pipeline35/1(q) -> correlator2/3(din1_im)
  pipeline36/1(q) -> correlator2/4(din2_re)
  pipeline37/1(q) -> correlator2/5(din2_im)
  pipeline38/1(q) -> correlator2/6(din_valid)
  centrosym3/1(y1_re) -> pipeline39/1(d)
  centrosym3/2(y1_im) -> pipeline40/1(d)
  centrosym3/3(y2_re) -> pipeline41/1(d)
  centrosym3/4(y2_im) -> pipeline42/1(d)
  centrosym3/5(dout_valid) -> pipeline43/1(d)
  pipeline39/1(q) -> correlator3/2(din1_re)
  pipeline40/1(q) -> correlator3/3(din1_im)
  pipeline41/1(q) -> correlator3/4(din2_re)
  pipeline42/1(q) -> correlator3/5(din2_im)
  pipeline43/1(q) -> correlator3/6(din_valid)
  correlator0/1(r11) -> corr_delay0/1(In1)
  correlator0/2(r22) -> corr_delay0/2(In2)
  correlator0/3(r12_re) -> corr_delay0/3(In3)
  correlator0/4(r12_im) -> corr_delay0/4(In4)
  correlator0/5(dout_valid) -> corr_delay0/5(valid_in)
  correlator1/1(r11) -> corr_delay1/1(In1)
  correlator1/2(r22) -> corr_delay1/2(In2)
  correlator1/3(r12_re) -> corr_delay1/3(In3)
  correlator1/4(r12_im) -> corr_delay1/4(In4)
  correlator1/5(dout_valid) -> corr_delay1/5(valid_in)
  correlator2/1(r11) -> corr_delay2/1(In1)
  correlator2/2(r22) -> corr_delay2/2(In2)
  correlator2/3(r12_re) -> corr_delay2/3(In3)
  correlator2/4(r12_im) -> corr_delay2/4(In4)
  correlator2/5(dout_valid) -> corr_delay2/5(valid_in)
  correlator3/1(r11) -> corr_delay3/1(In1)
  correlator3/2(r22) -> corr_delay3/2(In2)
  correlator3/3(r12_re) -> corr_delay3/3(In3)
  correlator3/4(r12_im) -> corr_delay3/4(In4)
  correlator3/5(dout_valid) -> corr_delay3/5(valid_in)
  corr_delay0/1(Out1) -> [r11_0]
  corr_delay0/2(Out2) -> [r22_0]
  corr_delay0/3(Out3) -> [r12_re_0]
  corr_delay0/5(valid_out) -> [corr_valid0]
  corr_delay1/1(Out1) -> [r11_1]
  corr_delay1/2(Out2) -> [r22_1]
  corr_delay1/3(Out3) -> [r12_re_1]
  corr_delay1/5(valid_out) -> [corr_valid1]
  corr_delay2/1(Out1) -> [r11_2]
  corr_delay2/2(Out2) -> [r22_2]
  corr_delay2/3(Out3) -> [r12_re_2]
  corr_delay2/5(valid_out) -> [corr_valid2]
  corr_delay3/1(Out1) -> [r11_3]
  corr_delay3/2(Out2) -> [r22_3]
  corr_delay3/3(Out3) -> [r12_re_3]
  corr_delay3/5(valid_out) -> [corr_valid3]
  doa_acc_len/1(in_reg) -> pipeline44/1(d)
  pipeline44/1(q) -> [doa_acc_len]
  Counter/1 -> r11/1(addr)
  Counter3/1 -> r12/1(addr)
  [doa_acc_len] -> acc_cntrl2/1(acc_len)
  [cnt_rst] -> acc_cntrl2/3(rst)
  [r11_3] -> Reinterpret/1
  [r11_2] -> Reinterpret5/1
  [r11_1] -> Reinterpret6/1
  [r11_0] -> Reinterpret7/1
  Concat/1 -> r11/2(data_in)
  Reinterpret/1 -> Concat/1
  Reinterpret5/1 -> Concat/2
  Reinterpret6/1 -> Concat/3
  Reinterpret7/1 -> Concat/4
  [corr_valid0] -> r11/3(we), Counter/2
  [cnt_rst] -> Counter/1
  [r12_3] -> Reinterpret8/1
  [r12_2] -> Reinterpret9/1
  [r12_1] -> Reinterpret10/1
  [r12_0] -> Reinterpret11/1
  Reinterpret8/1 -> Concat1/1
  Reinterpret9/1 -> Concat1/2
  Reinterpret10/1 -> Concat1/3
  Reinterpret11/1 -> Concat1/4
  Concat1/1 -> r12/2(data_in)
  [cnt_rst] -> Counter3/1
  [corr_valid0] -> r12/3(we), Counter3/2
  Counter4/1 -> r22/1
  [r22_3] -> Reinterpret14/1
  [r22_2] -> Reinterpret15/1
  [r22_1] -> Reinterpret12/1
  [r22_0] -> Reinterpret13/1
  Reinterpret14/1 -> Concat2/1
  Reinterpret15/1 -> Concat2/2
  Reinterpret12/1 -> Concat2/3
  Reinterpret13/1 -> Concat2/4
  Concat2/1 -> r22/2
  [cnt_rst] -> Counter4/1
  [corr_valid0] -> r22/3, Counter4/2
